simulink model "rfsoc_spectrometer"  (R2021a) (continued)
== system rfsoc_spectrometer == (continued)
      mask DialogControl t224_QT_adc0_AnalogSettings Prompt: Analog Settings
      mask DialogControl t224_QT_adc1 Prompt: ADC 1
      mask DialogControl t224_QT_adc1_DataSettings Prompt: Data Settings
      mask DialogControl t224_QT_adc1_req_axis_clk_label Prompt: Required AXI4-Stream clock:
      mask DialogControl t224_QT_adc1_MixerSettings Prompt: MIxer Settings
      mask DialogControl t224_QT_adc1_AnalogSettings Prompt: Analog Settings
      mask DialogControl ADCPair23 Prompt: ADC Pair 2,3
      mask DialogControl QT_adc2 Prompt: ADC 2
      mask DialogControl t224_QT_adc2_DataSettings Prompt: Data Settings
      mask DialogControl t224_QT_adc2_req_axis_clk_label Prompt: Required AXI4-Stream clock:
      mask DialogControl t224_QT_adc2_MixerSettings Prompt: MIxer Settings
      mask DialogControl t224_QT_adc2_AnalogSettings Prompt: Analog Settings
      mask DialogControl t224_QT_adc3 Prompt: ADC 3
      mask DialogControl t224_QT_adc3_DataSettings Prompt: Data Settings
      mask DialogControl t224_QT_adc3_req_axis_clk_label Prompt: Required AXI4-Stream clock:
      mask DialogControl t224_QT_adc3_MixerSettings Prompt: MIxer Settings
      mask DialogControl t224_QT_adc3_AnalogSettings Prompt: Analog Settings
      mask DialogControl t224_DualTileConfig Prompt: Dual Tile Configuration
      mask DialogControl t224_DT_adc0 Prompt: ADC 0
      mask DialogControl t224_DT_adc0_DataSettings Prompt: Data Settings
      mask DialogControl t224_DT_adc0_req_axis_clk_label Prompt: Required AXI4-Stream clock:
      mask DialogControl t224_DT_adc0_MixerSettings Prompt: MIxer Settings
      mask DialogControl t224_DT_adc0_AnalogSettings Prompt: Analog Settings
      mask DialogControl t224_DT_adc1 Prompt: ADC 1
      mask DialogControl t224_DT_adc1_DataSettings Prompt: Data Settings
      mask DialogControl t224_DT_adc1_req_axis_clk_label Prompt: Required AXI4-Stream clock:
      mask DialogControl t224_DT_adc1_MixerSettings Prompt: MIxer Settings
      mask DialogControl t224_DT_adc1_AnalogSettings Prompt: Analog Settings
      mask DialogControl Tile225_container Prompt: Tile 225
      mask DialogControl pll_clocking_config_225 Prompt: Clocking and PLL Configuration
      mask DialogControl t225_QuadTileConfig Prompt: Quad Tile Configuration
      mask DialogControl t225_ADCPair01 Prompt: ADC Pair 0,1
      mask DialogControl t225_QT_adc0 Prompt: ADC 0
      mask DialogControl t225_QT_adc0_DataSettings Prompt: Data Settings
      mask DialogControl t225_QT_adc0_req_axis_clk_label Prompt: Required AXI4-Stream clock:
      mask DialogControl t225_QT_adc0_MixerSettings Prompt: MIxer Settings
      mask DialogControl t225_QT_adc0_AnalogSettings Prompt: Analog Settings
      mask DialogControl t225_QT_adc1 Prompt: ADC 1
      mask DialogControl t225_QT_adc1_DataSettings Prompt: Data Settings
      mask DialogControl t225_QT_adc1_req_axis_clk_label Prompt: Required AXI4-Stream clock:
      mask DialogControl t225_QT_adc1_MixerSettings Prompt: MIxer Settings
      mask DialogControl t225_QT_adc1_AnalogSettings Prompt: Analog Settings
      mask DialogControl t225_ADCPair23 Prompt: ADC Pair 2,3
      mask DialogControl t225_QT_adc2 Prompt: ADC 2
      mask DialogControl t225_QT_adc2_DataSettings Prompt: Data Settings
      mask DialogControl t225_QT_adc2_req_axis_clk_label Prompt: Required AXI4-Stream clock:
      mask DialogControl t225_QT_adc2_MixerSettings Prompt: MIxer Settings
      mask DialogControl t225_QT_adc2_AnalogSettings Prompt: Analog Settings
      mask DialogControl t225_QT_adc3 Prompt: ADC 3
      mask DialogControl t225_QT_adc3_DataSettings Prompt: Data Settings
      mask DialogControl t225_QT_adc3_req_axis_clk_label Prompt: Required AXI4-Stream clock:
      mask DialogControl t225_QT_adc3_MixerSettings Prompt: MIxer Settings
      mask DialogControl t225_QT_adc3_AnalogSettings Prompt: Analog Settings
      mask DialogControl t225_DualTileConfig Prompt: Dual Tile Configuration
      mask DialogControl t225_DT_adc0 Prompt: ADC 0
      mask DialogControl t225_DT_adc0_DataSettings Prompt: Data Settings
      mask DialogControl t225_DT_adc0_req_axis_clk_label Prompt: Required AXI4-Stream clock:
      mask DialogControl t225_DT_adc0_MixerSettings Prompt: MIxer Settings
      mask DialogControl t225_DT_adc0_AnalogSettings Prompt: Analog Settings
      mask DialogControl t225_DT_adc1 Prompt: ADC 1
      mask DialogControl t225_DT_adc1_DataSettings Prompt: Data Settings
      mask DialogControl t225_DT_adc1_req_axis_clk_label Prompt: Required AXI4-Stream clock:
      mask DialogControl t225_DT_adc1_MixerSettings Prompt: MIxer Settings
      mask DialogControl t225_DT_adc1_AnalogSettings Prompt: Analog Settings
      mask DialogControl Tile226_container Prompt: Tile 226
      mask DialogControl pll_clocking_config_226 Prompt: Clocking and PLL Configuration
      mask DialogControl t226_QuadTileConfig Prompt: Quad Tile Configuration
      mask DialogControl t226_ADCPair01 Prompt: ADC Pair 0,1
      mask DialogControl t226_QT_adc0 Prompt: ADC 0
      mask DialogControl t226_QT_adc0_DataSettings Prompt: Data Settings
      mask DialogControl t226_QT_adc0_req_axis_clk_label Prompt: Required AXI4-Stream clock:
      mask DialogControl t226_QT_adc0_MixerSettings Prompt: MIxer Settings
      mask DialogControl t226_QT_adc0_AnalogSettings Prompt: Analog Settings
      mask DialogControl t226_QT_adc1 Prompt: ADC 1
      mask DialogControl t226_QT_adc1_DataSettings Prompt: Data Settings
      mask DialogControl t226_QT_adc1_req_axis_clk_label Prompt: Required AXI4-Stream clock:
      mask DialogControl t226_QT_adc1_MixerSettings Prompt: MIxer Settings
      mask DialogControl t226_QT_adc1_AnalogSettings Prompt: Analog Settings
      mask DialogControl t226_ADCPair23 Prompt: ADC Pair 2,3
      mask DialogControl t226_QT_adc2 Prompt: ADC 2
      mask DialogControl t226_QT_adc2_DataSettings Prompt: Data Settings
      mask DialogControl t226_QT_adc2_req_axis_clk_label Prompt: Required AXI4-Stream clock:
      mask DialogControl t226_QT_adc2_MixerSettings Prompt: MIxer Settings
      mask DialogControl t226_QT_adc2_AnalogSettings Prompt: Analog Settings
      mask DialogControl t226_QT_adc3 Prompt: ADC 3
      mask DialogControl t226_QT_adc3_DataSettings Prompt: Data Settings
      mask DialogControl t226_QT_adc3_req_axis_clk_label Prompt: Required AXI4-Stream clock:
      mask DialogControl t226_QT_adc3_MixerSettings Prompt: MIxer Settings
      mask DialogControl t226_QT_adc3_AnalogSettings Prompt: Analog Settings
      mask DialogControl t226_DualTileConfig Prompt: Dual Tile Configuration
      mask DialogControl t226_DT_adc0 Prompt: ADC 0
      mask DialogControl t226_DT_adc0_DataSettings Prompt: Data Settings
      mask DialogControl t226_DT_adc0_req_axis_clk_label Prompt: Required AXI4-Stream clock:
      mask DialogControl t226_DT_adc0_MixerSettings Prompt: MIxer Settings
      mask DialogControl t226_DT_adc0_AnalogSettings Prompt: Analog Settings
      mask DialogControl t226_DT_adc1 Prompt: ADC 1
      mask DialogControl t226_DT_adc1_DataSettings Prompt: Data Settings
      mask DialogControl t226_DT_adc1_req_axis_clk_label Prompt: Required AXI4-Stream clock:
      mask DialogControl t226_DT_adc1_MixerSettings Prompt: MIxer Settings
      mask DialogControl t226_DT_adc1_AnalogSettings Prompt: Analog Settings
      mask DialogControl Tile227_container Prompt: Tile 227
      mask DialogControl pll_clocking_config_227 Prompt: Clocking and PLL Configuration
      mask DialogControl t227_QuadTileConfig Prompt: Quad Tile Configuration
      mask DialogControl t227_ADCPair01 Prompt: ADC Pair 0,1
      mask DialogControl t227_QT_adc0 Prompt: ADC 0
      mask DialogControl t227_QT_adc0_DataSettings Prompt: Data Settings
      mask DialogControl t227_QT_adc0_req_axis_clk_label Prompt: Required AXI4-Stream clock:
      mask DialogControl t227_QT_adc0_MixerSettings Prompt: MIxer Settings
      mask DialogControl t227_QT_adc0_AnalogSettings Prompt: Analog Settings
      mask DialogControl t227_QT_adc1 Prompt: ADC 1
      mask DialogControl t227_QT_adc1_DataSettings Prompt: Data Settings
      mask DialogControl t227_QT_adc1_req_axis_clk_label Prompt: Required AXI4-Stream clock:
      mask DialogControl t227_QT_adc1_MixerSettings Prompt: MIxer Settings
      mask DialogControl t227_QT_adc1_AnalogSettings Prompt: Analog Settings
      mask DialogControl t227_ADCPair23 Prompt: ADC Pair 2,3
      mask DialogControl t227_QT_adc2 Prompt: ADC 2
      mask DialogControl t227_QT_adc2_DataSettings Prompt: Data Settings
      mask DialogControl t227_QT_adc2_req_axis_clk_label Prompt: Required AXI4-Stream clock:
      mask DialogControl t227_QT_adc2_MixerSettings Prompt: MIxer Settings
      mask DialogControl t227_QT_adc2_AnalogSettings Prompt: Analog Settings
      mask DialogControl t227_QT_adc3 Prompt: ADC 3
      mask DialogControl t227_QT_adc3_DataSettings Prompt: Data Settings
      mask DialogControl t227_QT_adc3_req_axis_clk_label Prompt: Required AXI4-Stream clock:
      mask DialogControl t227_QT_adc3_MixerSettings Prompt: MIxer Settings
      mask DialogControl t227_QT_adc3_AnalogSettings Prompt: Analog Settings
      mask DialogControl t227_DualTileConfig Prompt: Dual Tile Configuration
      mask DialogControl t227_DT_adc0 Prompt: ADC 0
      mask DialogControl t227_DT_adc0_DataSettings Prompt: Data Settings
      mask DialogControl t227_DT_adc0_req_axis_clk_label Prompt: Required AXI4-Stream clock:
      mask DialogControl t227_DT_adc0_MixerSettings Prompt: MIxer Settings
      mask DialogControl t227_DT_adc0_AnalogSettings Prompt: Analog Settings
      mask DialogControl t227_DT_adc1 Prompt: ADC 1
      mask DialogControl t227_DT_adc1_DataSettings Prompt: Data Settings
      mask DialogControl t227_DT_adc1_req_axis_clk_label Prompt: Required AXI4-Stream clock:
      mask DialogControl t227_DT_adc1_MixerSettings Prompt: MIxer Settings
      mask DialogControl t227_DT_adc1_AnalogSettings Prompt: Analog Settings
      mask DialogControl DACTileSelect Prompt: DAC Tiles
      mask DialogControl Tile228_container Prompt: Tile 228
      mask DialogControl pll_clocking_config_228 Prompt: Clocking and PLL Configuration
      mask DialogControl t228_QuadTileConfig Prompt: Quad Tile Configuration
      mask DialogControl t228_DACPair01 Prompt: DAC Pair 0,1
      mask DialogControl t228_QT_dac0 Prompt: DAC 0
      mask DialogControl t228_QT_dac0_DataSettings Prompt: Data Settings
      mask DialogControl t228_QT_dac0_req_axis_clk_label Prompt: Required AXI4-Stream clock:
      mask DialogControl t228_QT_dac0_MixerSettings Prompt: MIxer Settings
      mask DialogControl t228_QT_dac0_AnalogSettings Prompt: Analog Settings
      mask DialogControl t228_QT_dac1 Prompt: DAC 1
      mask DialogControl t228_QT_dac1_DataSettings Prompt: Data Settings
      mask DialogControl t228_QT_dac1_req_axis_clk_label Prompt: Required AXI4-Stream clock:
      mask DialogControl t228_QT_dac1_MixerSettings Prompt: MIxer Settings
      mask DialogControl t228_QT_dac1_AnalogSettings Prompt: Analog Settings
      mask DialogControl t228_DACPair23 Prompt: DAC Pair 2,3
      mask DialogControl t228_QT_dac2 Prompt: DAC 2
      mask DialogControl t228_QT_dac2_DataSettings Prompt: Data Settings
      mask DialogControl t228_QT_dac2_req_axis_clk_label Prompt: Required AXI4-Stream clock:
      mask DialogControl t228_QT_dac2_MixerSettings Prompt: MIxer Settings
      mask DialogControl t228_QT_dac2_AnalogSettings Prompt: Analog Settings
      mask DialogControl t228_QT_dac3 Prompt: DAC 3
      mask DialogControl t228_QT_dac3_DataSettings Prompt: Data Settings
      mask DialogControl t228_QT_dac3_req_axis_clk_label Prompt: Required AXI4-Stream clock:
      mask DialogControl t228_QT_dac3_MixerSettings Prompt: MIxer Settings
      mask DialogControl t228_QT_dac3_AnalogSettings Prompt: Analog Settings
      mask DialogControl t228_DualTileConfig Prompt: Dual Tile Configuration
      mask DialogControl t228_DACPair01_DT Prompt: DAC Pair 0,1
      mask DialogControl t228_DT_dac0 Prompt: DAC 0
      mask DialogControl t228_DT_dac0_DataSettings Prompt: Data Settings
      mask DialogControl t228_DT_dac0_req_axis_clk_label Prompt: Required AXI4-Stream clock:
      mask DialogControl t228_DT_dac0_MixerSettings Prompt: MIxer Settings
      mask DialogControl t228_DT_dac0_AnalogSettings Prompt: Analog Settings
      mask DialogControl t228_DT_dac1 Prompt: DAC 1
      mask DialogControl t228_DT_dac1_DataSettings Prompt: Data Settings
      mask DialogControl t228_DT_dac1_req_axis_clk_label Prompt: Required AXI4-Stream clock:
      mask DialogControl t228_DT_dac1_MixerSettings Prompt: MIxer Settings
      mask DialogControl t228_DT_dac1_AnalogSettings Prompt: Analog Settings
      mask DialogControl t228_DACPair23_DT Prompt: DAC Pair 2,3
      mask DialogControl t228_DT_dac2 Prompt: DAC 2
      mask DialogControl t228_DT_dac2_DataSettings Prompt: Data Settings
      mask DialogControl t228_DT_dac2_req_axis_clk_label Prompt: Required AXI4-Stream clock:
      mask DialogControl t228_DT_dac2_MixerSettings Prompt: MIxer Settings
      mask DialogControl t228_DT_dac2_AnalogSettings Prompt: Analog Settings
      mask DialogControl t228_DT_dac3 Prompt: DAC 3
      mask DialogControl t228_DT_dac3_DataSettings Prompt: Data Settings
      mask DialogControl t228_DT_dac3_req_axis_clk_label Prompt: Required AXI4-Stream clock:
      mask DialogControl t228_DT_dac3_MixerSettings Prompt: MIxer Settings
      mask DialogControl t228_DT_dac3_AnalogSettings Prompt: Analog Settings
      mask DialogControl Tile229_container Prompt: Tile 229
      mask DialogControl pll_clocking_config_229 Prompt: Clocking and PLL Configuration
      mask DialogControl t229_QuadTileConfig Prompt: Quad Tile Configuration
      mask DialogControl t229_DACPair01 Prompt: DAC Pair 0,1
      mask DialogControl t229_QT_dac0 Prompt: DAC 0
      mask DialogControl t229_QT_dac0_DataSettings Prompt: Data Settings
      mask DialogControl t229_QT_dac0_req_axis_clk_label Prompt: Required AXI4-Stream clock:
      mask DialogControl t229_QT_dac0_MixerSettings Prompt: MIxer Settings
      mask DialogControl t229_QT_dac0_AnalogSettings Prompt: Analog Settings
      mask DialogControl t229_QT_dac1 Prompt: DAC 1
      mask DialogControl t229_QT_dac1_DataSettings Prompt: Data Settings
      mask DialogControl t229_QT_dac1_req_axis_clk_label Prompt: Required AXI4-Stream clock:
      mask DialogControl t229_QT_dac1_MixerSettings Prompt: MIxer Settings
      mask DialogControl t229_QT_dac1_AnalogSettings Prompt: Analog Settings
      mask DialogControl t229_DACPair23 Prompt: DAC Pair 2,3
      mask DialogControl t229_QT_dac2 Prompt: DAC 2
      mask DialogControl t229_QT_dac2_DataSettings Prompt: Data Settings
      mask DialogControl t229_QT_dac2_req_axis_clk_label Prompt: Required AXI4-Stream clock:
      mask DialogControl t229_QT_dac2_MixerSettings Prompt: MIxer Settings
      mask DialogControl t229_QT_dac2_AnalogSettings Prompt: Analog Settings
      mask DialogControl t229_QT_dac3 Prompt: DAC 3
      mask DialogControl t229_QT_dac3_DataSettings Prompt: Data Settings
      mask DialogControl t229_QT_dac3_req_axis_clk_label Prompt: Required AXI4-Stream clock:
      mask DialogControl t229_QT_dac3_MixerSettings Prompt: MIxer Settings
      mask DialogControl t229_QT_dac3_AnalogSettings Prompt: Analog Settings
      mask DialogControl t229_DualTileConfig Prompt: Dual Tile Configuration
      mask DialogControl t229_DACPair01_DT Prompt: DAC Pair 0,1
      mask DialogControl t229_DT_dac0 Prompt: DAC 0
      mask DialogControl t229_DT_dac0_DataSettings Prompt: Data Settings
      mask DialogControl t229_DT_dac0_req_axis_clk_label Prompt: Required AXI4-Stream clock:
      mask DialogControl t229_DT_dac0_MixerSettings Prompt: MIxer Settings
      mask DialogControl t229_DT_dac0_AnalogSettings Prompt: Analog Settings
      mask DialogControl t229_DT_dac1 Prompt: DAC 1
      mask DialogControl t229_DT_dac1_DataSettings Prompt: Data Settings
      mask DialogControl t229_DT_dac1_req_axis_clk_label Prompt: Required AXI4-Stream clock:
      mask DialogControl t229_DT_dac1_MixerSettings Prompt: MIxer Settings
      mask DialogControl t229_DT_dac1_AnalogSettings Prompt: Analog Settings
      mask DialogControl t229_DACPair23_DT Prompt: DAC Pair 2,3
      mask DialogControl t229_DT_dac2 Prompt: DAC 2
      mask DialogControl t229_DT_dac2_DataSettings Prompt: Data Settings
      mask DialogControl t229_DT_dac2_req_axis_clk_label Prompt: Required AXI4-Stream clock:
      mask DialogControl t229_DT_dac2_MixerSettings Prompt: MIxer Settings
      mask DialogControl t229_DT_dac2_AnalogSettings Prompt: Analog Settings
      mask DialogControl t229_DT_dac3 Prompt: DAC 3
      mask DialogControl t229_DT_dac3_DataSettings Prompt: Data Settings
      mask DialogControl t229_DT_dac3_req_axis_clk_label Prompt: Required AXI4-Stream clock:
      mask DialogControl t229_DT_dac3_MixerSettings Prompt: MIxer Settings
      mask DialogControl t229_DT_dac3_AnalogSettings Prompt: Analog Settings
      mask DialogControl Tile230_container Prompt: Tile 230
      mask DialogControl pll_clocking_config_230 Prompt: Clocking and PLL Configuration
      mask DialogControl t230_QuadTileConfig Prompt: Quad Tile Configuration
      mask DialogControl t230_DACPair01 Prompt: DAC Pair 0,1
      mask DialogControl t230_QT_dac0 Prompt: DAC 0
      mask DialogControl t230_QT_dac0_DataSettings Prompt: Data Settings
      mask DialogControl t230_QT_dac0_req_axis_clk_label Prompt: Required AXI4-Stream clock:
      mask DialogControl t230_QT_dac0_MixerSettings Prompt: MIxer Settings
      mask DialogControl t230_QT_dac0_AnalogSettings Prompt: Analog Settings
      mask DialogControl t230_QT_dac1 Prompt: DAC 1
      mask DialogControl t230_QT_dac1_DataSettings Prompt: Data Settings
      mask DialogControl t230_QT_dac1_req_axis_clk_label Prompt: Required AXI4-Stream clock:
      mask DialogControl t230_QT_dac1_MixerSettings Prompt: MIxer Settings
      mask DialogControl t230_QT_dac1_AnalogSettings Prompt: Analog Settings
      mask DialogControl t230_DACPair23 Prompt: DAC Pair 2,3
      mask DialogControl t230_QT_dac2 Prompt: DAC 2
      mask DialogControl t230_QT_dac2_DataSettings Prompt: Data Settings
      mask DialogControl t230_QT_dac2_req_axis_clk_label Prompt: Required AXI4-Stream clock:
      mask DialogControl t230_QT_dac2_MixerSettings Prompt: MIxer Settings
      mask DialogControl t230_QT_dac2_AnalogSettings Prompt: Analog Settings
      mask DialogControl t230_QT_dac3 Prompt: DAC 3
      mask DialogControl t230_QT_dac3_DataSettings Prompt: Data Settings
      mask DialogControl t230_QT_dac3_req_axis_clk_label Prompt: Required AXI4-Stream clock:
      mask DialogControl t230_QT_dac3_MixerSettings Prompt: MIxer Settings
      mask DialogControl t230_QT_dac3_AnalogSettings Prompt: Analog Settings
      mask DialogControl t230_DualTileConfig Prompt: Dual Tile Configuration
      mask DialogControl Control58 Prompt: Tile 230 does not exist for DT configs
      mask DialogControl Tile231_container Prompt: Tile 231
      mask DialogControl pll_clocking_config_231 Prompt: Clocking and PLL Configuration
      mask DialogControl t231_QuadTileConfig Prompt: Quad Tile Configuration
      mask DialogControl t231_DACPair01 Prompt: DAC Pair 0,1
      mask DialogControl t231_QT_dac0 Prompt: DAC 0
      mask DialogControl t231_QT_dac0_DataSettings Prompt: Data Settings
      mask DialogControl t231_QT_dac0_req_axis_clk_label Prompt: Required AXI4-Stream clock:
      mask DialogControl t231_QT_dac0_MixerSettings Prompt: MIxer Settings
      mask DialogControl t231_QT_dac0_AnalogSettings Prompt: Analog Settings
      mask DialogControl t231_QT_dac1 Prompt: DAC 1
      mask DialogControl t231_QT_dac1_DataSettings Prompt: Data Settings
      mask DialogControl t231_QT_dac1_req_axis_clk_label Prompt: Required AXI4-Stream clock:
      mask DialogControl t231_QT_dac1_MixerSettings Prompt: MIxer Settings
      mask DialogControl t231_QT_dac1_AnalogSettings Prompt: Analog Settings
      mask DialogControl t231_DACPair23 Prompt: DAC Pair 2,3
      mask DialogControl t231_QT_dac2 Prompt: DAC 2
      mask DialogControl t231_QT_dac2_DataSettings Prompt: Data Settings
      mask DialogControl t231_QT_dac2_req_axis_clk_label Prompt: Required AXI4-Stream clock:
      mask DialogControl t231_QT_dac2_MixerSettings Prompt: MIxer Settings
      mask DialogControl t231_QT_dac2_AnalogSettings Prompt: Analog Settings
      mask DialogControl t231_QT_dac3 Prompt: DAC 3
      mask DialogControl t231_QT_dac3_DataSettings Prompt: Data Settings
      mask DialogControl t231_QT_dac3_req_axis_clk_label Prompt: Required AXI4-Stream clock:
      mask DialogControl t231_QT_dac3_MixerSettings Prompt: MIxer Settings
      mask DialogControl t231_QT_dac3_AnalogSettings Prompt: Analog Settings
      mask DialogControl t231_DualTileConfig Prompt: Dual Tile Configuration
      mask DialogControl Control59 Prompt: Tile 231 does not exist for DT configs
      mask DialogControl SystemClocking Prompt: System Clocking
      mask DialogControl ADCClocking Prompt: ADC Tile Clocking
      mask DialogControl t224_clock_config Prompt: ADC Tile 0
      mask DialogControl t225_clock_config Prompt: ADC Tile 1
      mask DialogControl t226_clock_config Prompt: ADC Tile 2
      mask DialogControl t227_clock_config Prompt: ADC Tile 3
      mask DialogControl DACClocking Prompt: DAC Tile Clocking
      mask DialogControl t228_clock_config Prompt: DAC Tile 0
      mask DialogControl t229_clock_config Prompt: DAC Tile 1
      mask DialogControl t230_clock_config Prompt: DAC Tile 2
      mask DialogControl t231_clock_config Prompt: DAC Tile 3
  snap_trig : Subsystem  Tag=xps:sw_reg  AncestorBlock=xps_library/Memory/software_register  mask(swreg){io_dir=From Processor, io_delay=0, init_val=dec2hex(0), sample_period=1, names=reg, bitwidths=32, bin_pts=0, arith_types=2, sim_port=off, show_format=on}
      mask prompts: io_dir "I/O direction"; io_delay "I/O delay"; init_val "Initial Value"; sample_period "Sample period"; names "Bitfield names [msb...lsb]"; bitwidths "Bitfield widths"; bin_pts "Bitfield binary pts"; arith_types "Bitfield types, ufix=0, fix=1, bool=2"; sim_port "Provide sim input/output?"; show_format "Print format string?"
      mask help: A 32-bit software-accessible register. Can be divided into bitfields of varying widths and types using the fields in the block mask.
      mask init:
      | swreg_init(gcb)
      settings: AttributesFormatString=1input:b = 32 bits
      mask TypeOptions io_dir: To Processor | From Processor
      mask Callback io_dir:
      | mask_enables = get_param(gcb, 'MaskEnables');
      | mask_names = get_param(gcb, 'MaskNames');
      | if strcmp(get_param(gcb, 'io_dir'), 'To Processor'),
      |     mask_enables{ismember(mask_names, 'sample_period')} = 'off';
      | else
      |     mask_enables{ismember(mask_names, 'sample_period')} = 'on';
      | end
      | set_param(gcb, 'MaskEnables', mask_enables);
      mask DialogControl ParameterTabVar0 Prompt: Setup
  snapshot_0 : Subsystem  Tag=casper:snapshot  AncestorBlock=casper_library_scopes/snapshot  mask(snapshot){storage=bram, dram_dimm=2, dram_clock=250, nsamples=12, data_width=128, offset=off, circap=off, value=off, ext_arm=off, ext_circ=off, provide_outputs=on, use_dsp48=off}
      mask prompts: storage "Storage medium"; dram_dimm "DRAM dimm"; dram_clock "DRAM clock rate"; nsamples "Number of Samples (2^?)"; data_width "Data width"; offset "Start delay support"; circap "Circular capture support"; value "Extra value capture support"; ext_arm "External arm support"; ext_circ "External circular capture support"; provide_outputs "Provide outputs"; use_dsp48 "Use DSP48s to implement counters"
      mask help:
      | Standard snap block, but with the following optional extras;
      |  1) Optionally delay the fabric trigger by a user-specified number of valids.
      |  2) Capture continuously after a trigger until a stop pulse is received. Data is captured into BRAM continuously, with old data being overwritten until a stop command is received. Then stop capturing when memory is filled. This enables you to get some data before and some data after the stop pulse. Use the 'tr_en_cnt' register to determine where you are in your vector. 'Addr' register's MSb now indicates 'busy capturing'.
      |  3) Optionally capture a value on an input port to a register at the same time as the first data sample.
      |  4) Implement counters using DSP48Es to save resources.
      mask init:
      | snapshot_init(gcb, ...
      |     'storage', storage, ...
      |     'dram_dimm', dram_dimm, ...
      |     'dram_clock', dram_clock, ...
      |     'nsamples', nsamples, ...
      |     'data_width', data_width, ...
      |     'offset', offset, ...
      |     'circap', circap, ...
      |     'value', value, ...
      |     'ext_arm', ext_arm, ...
      |     'ext_circ', ext_circ, ...
      |     'provide_outputs', 'off', ...
      |     'use_dsp48', use_dsp48);
      settings: AttributesFormatString=|
      | 128 wide, 4096 deep
      | debugID: 61751580
      mask TypeOptions storage: bram | dram
      mask Callback storage: snapshot_callback();
      mask TypeOptions dram_dimm: 1 | 2 | 3 | 4
      mask TypeOptions dram_clock: 200 | 250 | 266 | 300 | 333
      mask TypeOptions data_width: 8 | 16 | 32 | 64 | 128
  snapshot_1 : Subsystem  Tag=casper:snapshot  AncestorBlock=casper_library_scopes/snapshot  mask(snapshot){storage=bram, dram_dimm=2, dram_clock=250, nsamples=12, data_width=128, offset=off, circap=off, value=off, ext_arm=off, ext_circ=off, provide_outputs=on, use_dsp48=off}
      mask prompts: storage "Storage medium"; dram_dimm "DRAM dimm"; dram_clock "DRAM clock rate"; nsamples "Number of Samples (2^?)"; data_width "Data width"; offset "Start delay support"; circap "Circular capture support"; value "Extra value capture support"; ext_arm "External arm support"; ext_circ "External circular capture support"; provide_outputs "Provide outputs"; use_dsp48 "Use DSP48s to implement counters"
      mask help:
      | Standard snap block, but with the following optional extras;
      |  1) Optionally delay the fabric trigger by a user-specified number of valids.
      |  2) Capture continuously after a trigger until a stop pulse is received. Data is captured into BRAM continuously, with old data being overwritten until a stop command is received. Then stop capturing when memory is filled. This enables you to get some data before and some data after the stop pulse. Use the 'tr_en_cnt' register to determine where you are in your vector. 'Addr' register's MSb now indicates 'busy capturing'.
      |  3) Optionally capture a value on an input port to a register at the same time as the first data sample.
      |  4) Implement counters using DSP48Es to save resources.
      mask init: same as rfsoc_spectrometer/snapshot_0
      settings: AttributesFormatString=|
      | 128 wide, 4096 deep
      | debugID: 61751580
      mask TypeOptions storage: bram | dram
      mask Callback storage: snapshot_callback();
      mask TypeOptions dram_dimm: 1 | 2 | 3 | 4
      mask TypeOptions dram_clock: 200 | 250 | 266 | 300 | 333
      mask TypeOptions data_width: 8 | 16 | 32 | 64 | 128
  snapshot_2 : Subsystem  Tag=casper:snapshot  AncestorBlock=casper_library_scopes/snapshot  mask(snapshot){storage=bram, dram_dimm=2, dram_clock=250, nsamples=12, data_width=128, offset=off, circap=off, value=off, ext_arm=off, ext_circ=off, provide_outputs=on, use_dsp48=off}
      mask prompts: storage "Storage medium"; dram_dimm "DRAM dimm"; dram_clock "DRAM clock rate"; nsamples "Number of Samples (2^?)"; data_width "Data width"; offset "Start delay support"; circap "Circular capture support"; value "Extra value capture support"; ext_arm "External arm support"; ext_circ "External circular capture support"; provide_outputs "Provide outputs"; use_dsp48 "Use DSP48s to implement counters"
      mask help:
      | Standard snap block, but with the following optional extras;
      |  1) Optionally delay the fabric trigger by a user-specified number of valids.
      |  2) Capture continuously after a trigger until a stop pulse is received. Data is captured into BRAM continuously, with old data being overwritten until a stop command is received. Then stop capturing when memory is filled. This enables you to get some data before and some data after the stop pulse. Use the 'tr_en_cnt' register to determine where you are in your vector. 'Addr' register's MSb now indicates 'busy capturing'.
      |  3) Optionally capture a value on an input port to a register at the same time as the first data sample.
      |  4) Implement counters using DSP48Es to save resources.
      mask init: same as rfsoc_spectrometer/snapshot_0
      settings: AttributesFormatString=|
      | 128 wide, 4096 deep
      | debugID: 61751580
      mask TypeOptions storage: bram | dram
      mask Callback storage: snapshot_callback();
      mask TypeOptions dram_dimm: 1 | 2 | 3 | 4
      mask TypeOptions dram_clock: 200 | 250 | 266 | 300 | 333
      mask TypeOptions data_width: 8 | 16 | 32 | 64 | 128
  snapshot_3 : Subsystem  Tag=casper:snapshot  AncestorBlock=casper_library_scopes/snapshot  mask(snapshot){storage=bram, dram_dimm=2, dram_clock=250, nsamples=12, data_width=128, offset=off, circap=off, value=off, ext_arm=off, ext_circ=off, provide_outputs=on, use_dsp48=off}
      mask prompts: storage "Storage medium"; dram_dimm "DRAM dimm"; dram_clock "DRAM clock rate"; nsamples "Number of Samples (2^?)"; data_width "Data width"; offset "Start delay support"; circap "Circular capture support"; value "Extra value capture support"; ext_arm "External arm support"; ext_circ "External circular capture support"; provide_outputs "Provide outputs"; use_dsp48 "Use DSP48s to implement counters"
      mask help:
      | Standard snap block, but with the following optional extras;
      |  1) Optionally delay the fabric trigger by a user-specified number of valids.
      |  2) Capture continuously after a trigger until a stop pulse is received. Data is captured into BRAM continuously, with old data being overwritten until a stop command is received. Then stop capturing when memory is filled. This enables you to get some data before and some data after the stop pulse. Use the 'tr_en_cnt' register to determine where you are in your vector. 'Addr' register's MSb now indicates 'busy capturing'.
      |  3) Optionally capture a value on an input port to a register at the same time as the first data sample.
      |  4) Implement counters using DSP48Es to save resources.
      mask init: same as rfsoc_spectrometer/snapshot_0
      settings: AttributesFormatString=|
      | 128 wide, 4096 deep
      | debugID: 61751580
      mask TypeOptions storage: bram | dram
      mask Callback storage: snapshot_callback();
      mask TypeOptions dram_dimm: 1 | 2 | 3 | 4
      mask TypeOptions dram_clock: 200 | 250 | 266 | 300 | 333
      mask TypeOptions data_width: 8 | 16 | 32 | 64 | 128
  sync_gen : Subsystem  AncestorBlock=casper_library_misc/sync_gen  mask(sync_gen){sim_acc_len=1, fft_size=4096, fft_simult_inputs=8, pfb_fir_taps=3, reorder_vec=[2,2,9], scale=4096}
      mask prompts: sim_acc_len "Simulation Accumulation Length"; fft_size "FFT Size"; fft_simult_inputs "Simultaneous Inputs (FFT)"; pfb_fir_taps "Taps in pfb_fir (use 1 if no pfb_fir)"; reorder_vec "Reorder Orders (e.g. [2 2 2])"
      mask help:
      | This block computes the sync period based on system parameters as
      | described in Casper memo.  Detailed descritions of the block's
      | variables can be found in the 'Block Documentation' section of
      | the CASPER wiki.
      mask init:
      | sync_gen_init(gcb, ...
      |     'sim_acc_len', sim_acc_len, ...
      |     'fft_size', fft_size, ...
      |     'fft_simult_inputs', fft_simult_inputs,...
      |     'pfb_fir_taps', pfb_fir_taps, ...
      |     'reorder_vec', reorder_vec, ...
      |     'scale', scale);
      settings: AttributesFormatString=sim_sync_period=113246208
      mask Help:
      | SyncPeriod=LCM(reorder_vec)*(acc_num)*(pfb_fir_len)*(fft_size/fft_simult_inputs)
      | 
      | See CASPER sync pulse memo for more details.
  wires:
  rfdc/1(m00_axis_tdata) -> munge/1(din)
  [snap_trig] -> snapshot_0/3(trig)
  Constant2/1 -> rfdc/5(m20_axis_tdata_sim), rfdc/6(m22_axis_tdata_sim), rfdc/7(m30_axis_tdata_sim), rfdc/8(m32_axis_tdata_sim), rfdc/4(m12_axis_tdata_sim), rfdc/3(m10_axis_tdata_sim), rfdc/2(m02_axis_tdata_sim), rfdc/1(m00_axis_tdata_sim)
  snapshot_0/1(arm_out) -> Terminator/1
  snapshot_1/1(arm_out) -> Terminator8/1
  [snap_trig] -> snapshot_1/3(trig)
  rfdc/2(m02_axis_tdata) -> munge1/1(din)
  snap_trig/1(in_reg) -> [snap_trig]
  Constant1/1 -> snapshot_0/2(we)
  Constant3/1 -> snapshot_1/2(we)
  munge/1(dout) -> [m00]
  munge1/1(dout) -> [m02]
  munge2/1(dout) -> [m20]
  munge3/1(dout) -> [m22]
  rfdc/5(m20_axis_tdata) -> munge2/1(din)
  rfdc/6(m22_axis_tdata) -> munge3/1(din)
  [m00] -> snapshot_0/1(din), bus_expand1/1(bus_in)
  [m02] -> snapshot_1/1(din), bus_expand2/1(bus_in)
  [m20] -> snapshot_2/1(din), bus_expand3/1(bus_in)
  [snap_trig] -> snapshot_2/3(trig)
  Constant4/1 -> snapshot_2/2(we)
  snapshot_2/1(arm_out) -> Terminator1/1
  Constant5/1 -> snapshot_3/2(we)
  snapshot_3/1(arm_out) -> Terminator2/1
  [m22] -> snapshot_3/1(din), bus_expand4/1(bus_in)
  [snap_trig] -> snapshot_3/3(trig)
  bus_expand1/1(msb_out8) -> pfb_fir_real0/2(pol1_in1)
  bus_expand1/2(out7) -> pfb_fir_real0/3(pol1_in2)
  bus_expand1/3(out6) -> pfb_fir_real0/4(pol1_in3)
  bus_expand1/4(out5) -> pfb_fir_real0/5(pol1_in4)
  bus_expand1/5(out4) -> pfb_fir_real0/6(pol1_in5)
  bus_expand1/6(out3) -> pfb_fir_real0/7(pol1_in6)
  bus_expand1/7(out2) -> pfb_fir_real0/8(pol1_in7)
  bus_expand1/8(lsb_out1) -> pfb_fir_real0/9(pol1_in8)
  sync_gen/1(sync_out) -> pfb_fir_real1/1(sync), pfb_fir_real2/1(sync), pfb_fir_real3/1(sync), pfb_fir_real0/1(sync)
  pfb_fir_real0/1(sync_out) -> fft_wideband_real0/1(sync)
  pfb_fir_real0/2(pol1_out1) -> fft_wideband_real0/3(in00)
  pfb_fir_real0/3(pol1_out2) -> fft_wideband_real0/4(in01)
  pfb_fir_real0/4(pol1_out3) -> fft_wideband_real0/5(in02)
  pfb_fir_real0/5(pol1_out4) -> fft_wideband_real0/6(in03)
  pfb_fir_real0/6(pol1_out5) -> fft_wideband_real0/7(in04)
  pfb_fir_real0/7(pol1_out6) -> fft_wideband_real0/8(in05)
  pfb_fir_real0/8(pol1_out7) -> fft_wideband_real0/9(in06)
  pfb_fir_real0/9(pol1_out8) -> fft_wideband_real0/10(in07)
  fft_wideband_real0/1(sync_out) -> pre_power_delay0/1(sync_in)
  fft_wideband_real0/2(out00) -> pre_power_delay0/2(din0)
  fft_wideband_real0/3(out01) -> pre_power_delay0/3(din1)
  fft_wideband_real0/4(out02) -> pre_power_delay0/4(din2)
  fft_wideband_real0/5(out03) -> pre_power_delay0/5(din3)
  pre_power_delay0/1(sync_out) -> power0/1(sync_in)
  pre_power_delay0/2(dout0) -> power0/2(din0)
  pre_power_delay0/3(dout1) -> power0/3(din1)
  pre_power_delay0/4(dout2) -> power0/4(din2)
  pre_power_delay0/5(dout3) -> power0/5(din3)
  [cnt_rst] -> acc_cntrl0/3(rst)
  [acc_len] -> acc_cntrl0/1(acc_len)
  post_power_delay0/1(sync_out) -> acc_cntrl0/2(sync)
  pipeline8/1(q) -> [acc_len]
  acc_len/1(in_reg) -> pipeline8/1(d)
  pipeline1/1(q) -> [cnt_rst]
  cnt_rst/1(in_reg) -> pipeline1/1(d)
  acc_cntrl0/1(new_acc) -> counter0/1, accs0/1(new_acc)
  post_power_delay0/2(dout0) -> Delay/1
  post_power_delay0/3(dout1) -> Delay1/1
  post_power_delay0/5(dout3) -> Delay3/1
  post_power_delay0/4(dout2) -> Delay2/1
  Delay/1 -> accs0/2(din)
  Delay1/1 -> accs0/3(din1)
  Delay2/1 -> accs0/4(din2)
  Delay3/1 -> accs0/5(din3)
  accs0/1(valid) -> pre_bram_delay0/2(valid_in), counter0/2
  accs0/2(dout0) -> pre_bram_delay0/3(din0)
  accs0/3(dout1) -> pre_bram_delay0/4(din1)
  accs0/4(dout2) -> pre_bram_delay0/5(din2)
  accs0/5(dout3) -> pre_bram_delay0/6(din3)
  counter0/1 -> pre_bram_delay0/1(addr_in)
  pre_bram_delay0/1(addr_out) -> adc0_2/1(addr), adc0_3/1(addr), adc0_1/1(addr), adc0_0/1(addr)
  pre_bram_delay0/3(dout0) -> adc0_0/2(data_in)
  pre_bram_delay0/2(valid_out) -> adc0_3/3(we), adc0_2/3(we), adc0_1/3(we), adc0_0/3(we)
  pre_bram_delay0/4(dout1) -> adc0_1/2(data_in)
  pre_bram_delay0/5(dout2) -> adc0_2/2(data_in)
  pre_bram_delay0/6(dout3) -> adc0_3/2(data_in)
  adc0_0/1(data_out) -> Terminator3/1
  adc0_1/1(data_out) -> Terminator4/1
  adc0_2/1(data_out) -> Terminator5/1
  adc0_3/1(data_out) -> Terminator6/1
  post_power_delay1/1(sync_out) -> acc_cntrl1/2(sync)
  pfb_fir_real1/2(pol1_out1) -> fft_wideband_real1/3(in00)
  [cnt_rst] -> acc_cntrl1/3(rst)
  pfb_fir_real1/6(pol1_out5) -> fft_wideband_real1/7(in04)
  Delay7/1 -> accs1/5(din3)
  pre_bram_delay1/5(dout2) -> adc1_2/2(data_in)
  adc1_1/1(data_out) -> Terminator9/1
  accs1/1(valid) -> counter1/2, pre_bram_delay1/2(valid_in)
  Delay5/1 -> accs1/3(din1)
  [acc_len] -> acc_cntrl1/1(acc_len)
  pfb_fir_real1/8(pol1_out7) -> fft_wideband_real1/9(in06)
  accs1/3(dout1) -> pre_bram_delay1/4(din1)
  post_power_delay1/2(dout0) -> Delay4/1
  fft_wideband_real1/5(out03) -> pre_power_delay1/5(din3)
  pre_power_delay1/4(dout2) -> power1/4(din2)
  pfb_fir_real1/4(pol1_out3) -> fft_wideband_real1/5(in02)
  [fft_shift] -> fft_wideband_real1/2(shift)
  pfb_fir_real1/1(sync_out) -> fft_wideband_real1/1(sync)
  pre_bram_delay1/2(valid_out) -> adc1_2/3(we), adc1_3/3(we), adc1_1/3(we), adc1_0/3(we)
  pre_bram_delay1/4(dout1) -> adc1_1/2(data_in)
  Delay4/1 -> accs1/2(din)
  accs1/5(dout3) -> pre_bram_delay1/6(din3)
  fft_wideband_real1/3(out01) -> pre_power_delay1/3(din1)
  pre_power_delay1/2(dout0) -> power1/2(din0)
  Delay6/1 -> accs1/4(din2)
  fft_wideband_real1/4(out02) -> pre_power_delay1/4(din2)
  pfb_fir_real1/7(pol1_out6) -> fft_wideband_real1/8(in05)
  pre_power_delay1/1(sync_out) -> power1/1(sync_in)
  post_power_delay1/5(dout3) -> Delay7/1
  fft_wideband_real1/1(sync_out) -> pre_power_delay1/1(sync_in)
  pre_bram_delay1/6(dout3) -> adc1_3/2(data_in)
  pfb_fir_real1/9(pol1_out8) -> fft_wideband_real1/10(in07)
  pre_bram_delay1/1(addr_out) -> adc1_2/1(addr), adc1_3/1(addr), adc1_1/1(addr), adc1_0/1(addr)
  acc_cntrl1/1(new_acc) -> counter1/1, accs1/1(new_acc)
  fft_wideband_real1/2(out00) -> pre_power_delay1/2(din0)
  pfb_fir_real1/5(pol1_out4) -> fft_wideband_real1/6(in03)
  pfb_fir_real1/3(pol1_out2) -> fft_wideband_real1/4(in01)
  pre_power_delay1/5(dout3) -> power1/5(din3)
  counter1/1 -> pre_bram_delay1/1(addr_in)
  pre_bram_delay1/3(dout0) -> adc1_0/2(data_in)
  accs1/4(dout2) -> pre_bram_delay1/5(din2)
  post_power_delay1/4(dout2) -> Delay6/1
  post_power_delay1/3(dout1) -> Delay5/1
  adc1_0/1(data_out) -> Terminator7/1
  pre_power_delay1/3(dout1) -> power1/3(din1)
  accs1/2(dout0) -> pre_bram_delay1/3(din0)
  adc1_3/1(data_out) -> Terminator11/1
  adc1_2/1(data_out) -> Terminator10/1
  bus_expand2/1(msb_out8) -> pfb_fir_real1/2(pol1_in1)
  bus_expand2/2(out7) -> pfb_fir_real1/3(pol1_in2)
  bus_expand2/3(out6) -> pfb_fir_real1/4(pol1_in3)
  bus_expand2/4(out5) -> pfb_fir_real1/5(pol1_in4)
  bus_expand2/5(out4) -> pfb_fir_real1/6(pol1_in5)
  bus_expand2/6(out3) -> pfb_fir_real1/7(pol1_in6)
  bus_expand2/7(out2) -> pfb_fir_real1/8(pol1_in7)
  bus_expand2/8(lsb_out1) -> pfb_fir_real1/9(pol1_in8)
  post_power_delay2/1(sync_out) -> acc_cntrl2/2(sync)
  pfb_fir_real2/2(pol1_out1) -> fft_wideband_real2/3(in00)
  [cnt_rst] -> acc_cntrl2/3(rst)
  pfb_fir_real2/6(pol1_out5) -> fft_wideband_real2/7(in04)
  Delay11/1 -> accs2/5(din3)
  pre_bram_delay2/5(dout2) -> adc2_2/2(data_in)
  adc2_1/1(data_out) -> Terminator13/1
  accs2/1(valid) -> counter2/2, pre_bram_delay2/2(valid_in)
  Delay9/1 -> accs2/3(din1)
  [acc_len] -> acc_cntrl2/1(acc_len)
  pfb_fir_real2/8(pol1_out7) -> fft_wideband_real2/9(in06)
  accs2/3(dout1) -> pre_bram_delay2/4(din1)
  post_power_delay2/2(dout0) -> Delay8/1
  fft_wideband_real2/5(out03) -> pre_power_delay2/5(din3)
  pre_power_delay2/4(dout2) -> power2/4(din2)
  pfb_fir_real2/4(pol1_out3) -> fft_wideband_real2/5(in02)
  [fft_shift] -> fft_wideband_real2/2(shift)
  pfb_fir_real2/1(sync_out) -> fft_wideband_real2/1(sync)
  pre_bram_delay2/2(valid_out) -> adc2_2/3(we), adc2_3/3(we), adc2_1/3(we), adc2_0/3(we)
  pre_bram_delay2/4(dout1) -> adc2_1/2(data_in)
  Delay8/1 -> accs2/2(din)
  accs2/5(dout3) -> pre_bram_delay2/6(din3)
  fft_wideband_real2/3(out01) -> pre_power_delay2/3(din1)
  pre_power_delay2/2(dout0) -> power2/2(din0)
  Delay10/1 -> accs2/4(din2)
  fft_wideband_real2/4(out02) -> pre_power_delay2/4(din2)
  pfb_fir_real2/7(pol1_out6) -> fft_wideband_real2/8(in05)
  pre_power_delay2/1(sync_out) -> power2/1(sync_in)
  post_power_delay2/5(dout3) -> Delay11/1
  fft_wideband_real2/1(sync_out) -> pre_power_delay2/1(sync_in)
  pre_bram_delay2/6(dout3) -> adc2_3/2(data_in)
  pfb_fir_real2/9(pol1_out8) -> fft_wideband_real2/10(in07)
  pre_bram_delay2/1(addr_out) -> adc2_2/1(addr), adc2_3/1(addr), adc2_1/1(addr), adc2_0/1(addr)
  acc_cntrl2/1(new_acc) -> accs2/1(new_acc), counter2/1
  fft_wideband_real2/2(out00) -> pre_power_delay2/2(din0)
  pfb_fir_real2/5(pol1_out4) -> fft_wideband_real2/6(in03)
  pfb_fir_real2/3(pol1_out2) -> fft_wideband_real2/4(in01)
  pre_power_delay2/5(dout3) -> power2/5(din3)
  counter2/1 -> pre_bram_delay2/1(addr_in)
  pre_bram_delay2/3(dout0) -> adc2_0/2(data_in)
  accs2/4(dout2) -> pre_bram_delay2/5(din2)
  post_power_delay2/4(dout2) -> Delay10/1
  post_power_delay2/3(dout1) -> Delay9/1
  adc2_0/1(data_out) -> Terminator12/1
  pre_power_delay2/3(dout1) -> power2/3(din1)
  accs2/2(dout0) -> pre_bram_delay2/3(din0)
  adc2_3/1(data_out) -> Terminator15/1
  adc2_2/1(data_out) -> Terminator14/1
  bus_expand3/1(msb_out8) -> pfb_fir_real2/2(pol1_in1)
  bus_expand3/2(out7) -> pfb_fir_real2/3(pol1_in2)
  bus_expand3/3(out6) -> pfb_fir_real2/4(pol1_in3)
  bus_expand3/4(out5) -> pfb_fir_real2/5(pol1_in4)
  bus_expand3/5(out4) -> pfb_fir_real2/6(pol1_in5)
  bus_expand3/6(out3) -> pfb_fir_real2/7(pol1_in6)
  bus_expand3/7(out2) -> pfb_fir_real2/8(pol1_in7)
  bus_expand3/8(lsb_out1) -> pfb_fir_real2/9(pol1_in8)
  post_power_delay3/1(sync_out) -> acc_cntrl3/2(sync)
  pfb_fir_real3/2(pol1_out1) -> fft_wideband_real3/3(in00)
  [cnt_rst] -> acc_cntrl3/3(rst)
  pfb_fir_real3/6(pol1_out5) -> fft_wideband_real3/7(in04)
  Delay15/1 -> accs3/5(din3)
  pre_bram_delay3/5(dout2) -> adc3_2/2(data_in)
  adc3_1/1(data_out) -> Terminator17/1
  accs3/1(valid) -> counter3/2, pre_bram_delay3/2(valid_in)
  Delay13/1 -> accs3/3(din1)
  [acc_len] -> acc_cntrl3/1(acc_len)
  pfb_fir_real3/8(pol1_out7) -> fft_wideband_real3/9(in06)
  accs3/3(dout1) -> pre_bram_delay3/4(din1)
  post_power_delay3/2(dout0) -> Delay12/1
  fft_wideband_real3/5(out03) -> pre_power_delay3/5(din3)
  pre_power_delay3/4(dout2) -> power3/4(din2)
  pfb_fir_real3/4(pol1_out3) -> fft_wideband_real3/5(in02)
  [fft_shift] -> fft_wideband_real3/2(shift)
  pfb_fir_real3/1(sync_out) -> fft_wideband_real3/1(sync)
  pre_bram_delay3/2(valid_out) -> adc3_2/3(we), adc3_3/3(we), adc3_1/3(we), adc3_0/3(we)
  pre_bram_delay3/4(dout1) -> adc3_1/2(data_in)
  Delay12/1 -> accs3/2(din)
  accs3/5(dout3) -> pre_bram_delay3/6(din3)
  fft_wideband_real3/3(out01) -> pre_power_delay3/3(din1)
  pre_power_delay3/2(dout0) -> power3/2(din0)
  Delay14/1 -> accs3/4(din2)
  fft_wideband_real3/4(out02) -> pre_power_delay3/4(din2)
  pfb_fir_real3/7(pol1_out6) -> fft_wideband_real3/8(in05)
  pre_power_delay3/1(sync_out) -> power3/1(sync_in)
  post_power_delay3/5(dout3) -> Delay15/1
  fft_wideband_real3/1(sync_out) -> pre_power_delay3/1(sync_in)
  pre_bram_delay3/6(dout3) -> adc3_3/2(data_in)
  pfb_fir_real3/9(pol1_out8) -> fft_wideband_real3/10(in07)
  pre_bram_delay3/1(addr_out) -> adc3_3/1(addr), adc3_2/1(addr), adc3_1/1(addr), adc3_0/1(addr)
  acc_cntrl3/1(new_acc) -> counter3/1, accs3/1(new_acc)
  fft_wideband_real3/2(out00) -> pre_power_delay3/2(din0)
  pfb_fir_real3/5(pol1_out4) -> fft_wideband_real3/6(in03)
  pfb_fir_real3/3(pol1_out2) -> fft_wideband_real3/4(in01)
  pre_power_delay3/5(dout3) -> power3/5(din3)
  counter3/1 -> pre_bram_delay3/1(addr_in)
  pre_bram_delay3/3(dout0) -> adc3_0/2(data_in)
  accs3/4(dout2) -> pre_bram_delay3/5(din2)
  post_power_delay3/4(dout2) -> Delay14/1
  post_power_delay3/3(dout1) -> Delay13/1
  adc3_0/1(data_out) -> Terminator16/1
  pre_power_delay3/3(dout1) -> power3/3(din1)
  accs3/2(dout0) -> pre_bram_delay3/3(din0)
  adc3_3/1(data_out) -> Terminator19/1
  adc3_2/1(data_out) -> Terminator18/1
  bus_expand4/1(msb_out8) -> pfb_fir_real3/2(pol1_in1)
  bus_expand4/2(out7) -> pfb_fir_real3/3(pol1_in2)
  bus_expand4/3(out6) -> pfb_fir_real3/4(pol1_in3)
  bus_expand4/4(out5) -> pfb_fir_real3/5(pol1_in4)
  bus_expand4/5(out4) -> pfb_fir_real3/6(pol1_in5)
  bus_expand4/6(out3) -> pfb_fir_real3/7(pol1_in6)
  bus_expand4/7(out2) -> pfb_fir_real3/8(pol1_in7)
  bus_expand4/8(lsb_out1) -> pfb_fir_real3/9(pol1_in8)
  power0/1(sync_out) -> power_convert0/1(sync_in)
  power0/2(dout0) -> power_convert0/2(din0)
  power0/3(dout1) -> power_convert0/3(din1)
  power0/4(dout2) -> power_convert0/4(din2)
  power0/5(dout3) -> power_convert0/5(din3)
  power_convert0/1(sync_out) -> post_power_delay0/1(sync_in)
  power_convert0/2(dout0) -> post_power_delay0/2(din0)
  power_convert0/3(dout1) -> post_power_delay0/3(din1)
  power_convert0/4(dout2) -> post_power_delay0/4(din2)
  power_convert0/5(dout3) -> post_power_delay0/5(din3)
  power1/1(sync_out) -> power_convert1/1(sync_in)
  power1/2(dout0) -> power_convert1/2(din0)
  power1/3(dout1) -> power_convert1/3(din1)
  power1/4(dout2) -> power_convert1/4(din2)
  power1/5(dout3) -> power_convert1/5(din3)
  power_convert1/1(sync_out) -> post_power_delay1/1(sync_in)
  power_convert1/2(dout0) -> post_power_delay1/2(din0)
  power_convert1/3(dout1) -> post_power_delay1/3(din1)
  power_convert1/4(dout2) -> post_power_delay1/4(din2)
  power_convert1/5(dout3) -> post_power_delay1/5(din3)
  power2/1(sync_out) -> power_convert2/1(sync_in)
  power2/2(dout0) -> power_convert2/2(din0)
  power2/3(dout1) -> power_convert2/3(din1)
  power2/4(dout2) -> power_convert2/4(din2)
  power2/5(dout3) -> power_convert2/5(din3)
  power_convert2/1(sync_out) -> post_power_delay2/1(sync_in)
  power_convert2/2(dout0) -> post_power_delay2/2(din0)
  power_convert2/3(dout1) -> post_power_delay2/3(din1)
  power_convert2/4(dout2) -> post_power_delay2/4(din2)
  power_convert2/5(dout3) -> post_power_delay2/5(din3)
  power3/1(sync_out) -> power_convert3/1(sync_in)
  power3/2(dout0) -> power_convert3/2(din0)
  power3/3(dout1) -> power_convert3/3(din1)
  power3/4(dout2) -> power_convert3/4(din2)
  power3/5(dout3) -> power_convert3/5(din3)
  power_convert3/1(sync_out) -> post_power_delay3/1(sync_in)
  power_convert3/2(dout0) -> post_power_delay3/2(din0)
  power_convert3/3(dout1) -> post_power_delay3/3(din1)
  power_convert3/4(dout2) -> post_power_delay3/4(din2)
  power_convert3/5(dout3) -> post_power_delay3/5(din3)
  Constant6/1 -> [fft_shift]
  [fft_shift] -> fft_wideband_real0/2(shift)
